simulink model "CCMVgaMotor"  (R2022b)
settings: Solver=FixedStepDiscrete  StartTime=0.0  StopTime=10.0  FixedStep=0.01  RelTol=1e-3
model workspace (MAT data in the model file):
  NvMBlockVgaMtrCalData : Simulink.Signal (value not decoded)
  NvMBlockVgaMtrCalDataInit : Simulink.Parameter (value not decoded)
  VgaWakeUpFlag : Simulink.Signal (value not decoded)
symbols that are objects in the model workspace (value not decoded): NvMBlockVgaMtrCalDataInit
undefined symbols (not defined in the file or in its folder): ComChlType, DioChannelType, LinFrameType, NetworkChlType, NvMBlockId, P_DioLevelType, P_LinIfSchHandleType, P_Std_ReturnType, PowerChlType, VGAChlType
(part 2 of 4)

== system CCMVgaMotor/CCMVGAMotor_VentnMotAdr2_sys ==
  function : TriggerPort  TriggerType=function-call  VariantControl=(inherit)
  PA_NvMBlockVgaMtrCalDataInit : Constant  Value=NvMBlockVgaMtrCalDataInit
  Terminator_1 : Terminator
  wires:
  PA_NvMBlockVgaMtrCalDataInit/1 -> Terminator_1/1

== system CCMVgaMotor/CCMVGAMotor_VentnMotAdr_sys ==
  function : TriggerPort  TriggerType=function-call  VariantControl=(inherit)
  PA_NvMBlockVgaMtrCalDataInit : Constant  Value=NvMBlockVgaMtrCalDataInit
  Terminator_1 : Terminator
  wires:
  PA_NvMBlockVgaMtrCalDataInit/1 -> Terminator_1/1

== system CCMVgaMotor/CCMVgaMotor_50msRunnable_sys ==
  function : TriggerPort  TriggerType=function-call  VariantControl=(inherit)  SampleTimeType=periodic  SampleTime=-1
  PA_NvMBlockVgaMtrCalDataInit : Constant  Value=NvMBlockVgaMtrCalDataInit
  Terminator_1 : Terminator
  wires:
  PA_NvMBlockVgaMtrCalDataInit/1 -> Terminator_1/1

== system CCMVgaMotor/CCMVgaMotor_Init ==
  SbcWakeupSta_SbcWakeupSta_read : Inport
  SbcPwrOn_SbcPwrOn_read : Inport  Port=2
  Event Listener : EventListener  EventName=reset
  PA_NvMBlockVgaMtrCalDataInit : Constant  Value=NvMBlockVgaMtrCalDataInit
  Terminator_1 : Terminator
  Terminator_2 : Terminator
  Terminator_3 : Terminator
  wires:
  SbcWakeupSta_SbcWakeupSta_read/1 -> Terminator_1/1
  SbcPwrOn_SbcPwrOn_read/1 -> Terminator_2/1
  PA_NvMBlockVgaMtrCalDataInit/1 -> Terminator_3/1
